simulink model "two_adcs_rfsoc_if_calibrator"  (R2021a)
settings: Solver=VariableStepAuto  StartTime=0.0  StopTime=10.0  RelTol=1e-3
undefined symbols (not defined in the file or in its folder): pfb_coeff_gen_calc
libraries: xbsIndex_r4 x30262, casper_library_misc x256, casper_library_delays x105, xps_library x1
(part 13 of 283)

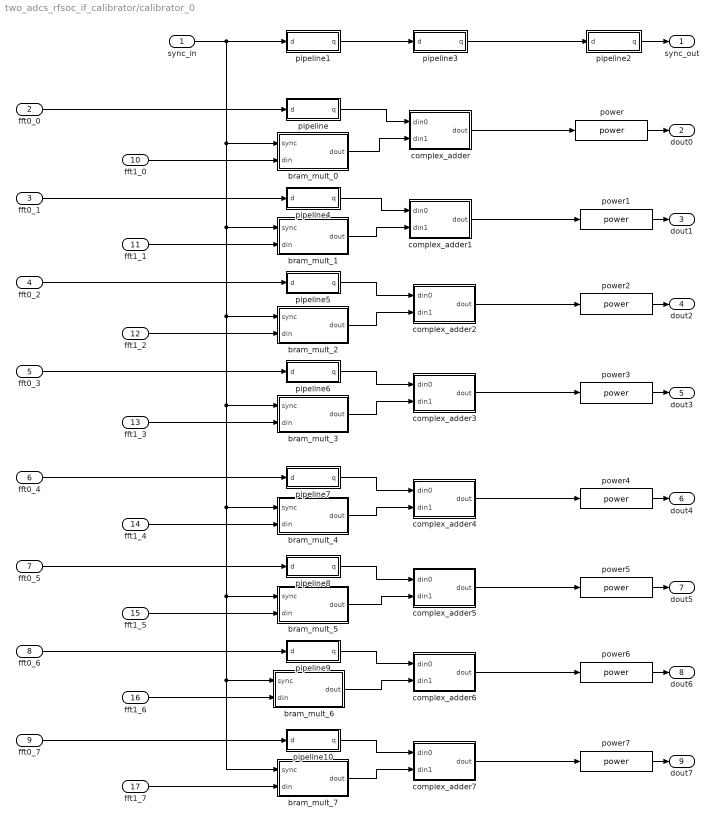
== system two_adcs_rfsoc_if_calibrator/calibrator_0 ==
  sync_in : Inport
  fft0_0 : Inport  Port=2
  fft0_1 : Inport  Port=3
  fft0_2 : Inport  Port=4
  fft0_3 : Inport  Port=5
  fft0_4 : Inport  Port=6
  fft0_5 : Inport  Port=7
  fft0_6 : Inport  Port=8
  fft0_7 : Inport  Port=9
  fft1_0 : Inport  Port=10
  fft1_1 : Inport  Port=11
  fft1_2 : Inport  Port=12
  fft1_3 : Inport  Port=13
  fft1_4 : Inport  Port=14
  fft1_5 : Inport  Port=15
  fft1_6 : Inport  Port=16
  fft1_7 : Inport  Port=17
  bram_mult_0 : Subsystem  mask{din_width=din_width, din_point=din_point, bram_width=bram_width, bram_point=bram_point, log2_vec_len=log2_vec_len, dout_width=din_width, dout_point=din_point}
      mask prompts: din_width "din width"; din_point "din point"; bram_width "bram width"; log2_vec_len "vector len (2?)"; dout_width "dout width"; dout_point "dout point"
      mask init:
      | name = gcb();
      | bram_name = strcat(name, '/bram_re');
      | set_param(bram_name, 'data_width', int2str(bram_width));
      | bram_name = strcat(name, '/bram_im');
      | set_param(bram_name, 'data_width', int2str(bram_width));
      mask Display:
      | port_label('input', 1, 'sync');
      | port_label('input', 2, 'din');
      | port_label('output', 1, 'dout');
      | disp('Z^-5')
  bram_mult_1 : Subsystem  mask{din_width=din_width, din_point=din_point, bram_width=bram_width, bram_point=bram_point, log2_vec_len=log2_vec_len, dout_width=din_width, dout_point=din_point}
      mask prompts: din_width "din width"; din_point "din point"; bram_width "bram width"; log2_vec_len "vector len (2?)"; dout_width "dout width"; dout_point "dout point"
      mask init: same as two_adcs_rfsoc_if_calibrator/calibrator_0/bram_mult_0
      mask Display:
      | port_label('input', 1, 'sync');
      | port_label('input', 2, 'din');
      | port_label('output', 1, 'dout');
      | disp('Z^-5')
  bram_mult_2 : Subsystem  mask{din_width=din_width, din_point=din_point, bram_width=bram_width, bram_point=bram_point, log2_vec_len=log2_vec_len, dout_width=din_width, dout_point=din_point}
      mask prompts: din_width "din width"; din_point "din point"; bram_width "bram width"; log2_vec_len "vector len (2?)"; dout_width "dout width"; dout_point "dout point"
      mask init: same as two_adcs_rfsoc_if_calibrator/calibrator_0/bram_mult_0
      mask Display:
      | port_label('input', 1, 'sync');
      | port_label('input', 2, 'din');
      | port_label('output', 1, 'dout');
      | disp('Z^-5')
  bram_mult_3 : Subsystem  mask{din_width=din_width, din_point=din_point, bram_width=bram_width, bram_point=bram_point, log2_vec_len=log2_vec_len, dout_width=din_width, dout_point=din_point}
      mask prompts: din_width "din width"; din_point "din point"; bram_width "bram width"; log2_vec_len "vector len (2?)"; dout_width "dout width"; dout_point "dout point"
      mask init: same as two_adcs_rfsoc_if_calibrator/calibrator_0/bram_mult_0
      mask Display:
      | port_label('input', 1, 'sync');
      | port_label('input', 2, 'din');
      | port_label('output', 1, 'dout');
      | disp('Z^-5')
  bram_mult_4 : Subsystem  mask{din_width=din_width, din_point=din_point, bram_width=bram_width, bram_point=bram_point, log2_vec_len=log2_vec_len, dout_width=din_width, dout_point=din_point}
      mask prompts: din_width "din width"; din_point "din point"; bram_width "bram width"; log2_vec_len "vector len (2?)"; dout_width "dout width"; dout_point "dout point"
      mask init: same as two_adcs_rfsoc_if_calibrator/calibrator_0/bram_mult_0
      mask Display:
      | port_label('input', 1, 'sync');
      | port_label('input', 2, 'din');
      | port_label('output', 1, 'dout');
      | disp('Z^-5')
  bram_mult_5 : Subsystem  mask{din_width=din_width, din_point=din_point, bram_width=bram_width, bram_point=bram_point, log2_vec_len=log2_vec_len, dout_width=din_width, dout_point=din_point}
      mask prompts: din_width "din width"; din_point "din point"; bram_width "bram width"; log2_vec_len "vector len (2?)"; dout_width "dout width"; dout_point "dout point"
      mask init: same as two_adcs_rfsoc_if_calibrator/calibrator_0/bram_mult_0
      mask Display:
      | port_label('input', 1, 'sync');
      | port_label('input', 2, 'din');
      | port_label('output', 1, 'dout');
      | disp('Z^-5')
  bram_mult_6 : Subsystem  mask{din_width=din_width, din_point=din_point, bram_width=bram_width, bram_point=bram_point, log2_vec_len=log2_vec_len, dout_width=din_width, dout_point=din_point}
      mask prompts: din_width "din width"; din_point "din point"; bram_width "bram width"; log2_vec_len "vector len (2?)"; dout_width "dout width"; dout_point "dout point"
      mask init: same as two_adcs_rfsoc_if_calibrator/calibrator_0/bram_mult_0
      mask Display:
      | port_label('input', 1, 'sync');
      | port_label('input', 2, 'din');
      | port_label('output', 1, 'dout');
      | disp('Z^-5')
  bram_mult_7 : Subsystem  mask{din_width=din_width, din_point=din_point, bram_width=bram_width, bram_point=bram_point, log2_vec_len=log2_vec_len, dout_width=din_width, dout_point=din_point}
      mask prompts: din_width "din width"; din_point "din point"; bram_width "bram width"; log2_vec_len "vector len (2?)"; dout_width "dout width"; dout_point "dout point"
      mask init: same as two_adcs_rfsoc_if_calibrator/calibrator_0/bram_mult_0
      mask Display:
      | port_label('input', 1, 'sync');
      | port_label('input', 2, 'din');
      | port_label('output', 1, 'dout');
      | disp('Z^-5')
  complex_adder : Subsystem  mask{Parameter1=din_width, din_point=din_point, dout_width=din_width, dout_point=din_point, add_lat=2, conv_lat=1}
      mask prompts: Parameter1 "din width"; din_point "din point"; dout_width "dout width"; dout_point "dout point"; add_lat "add delay"; conv_lat "conv delay"
  complex_adder1 : Subsystem  mask{Parameter1=din_width, din_point=din_point, dout_width=din_width, dout_point=din_point, add_lat=2, conv_lat=1}
      mask prompts: Parameter1 "din width"; din_point "din point"; dout_width "dout width"; dout_point "dout point"; add_lat "add delay"; conv_lat "conv delay"
  complex_adder2 : Subsystem  mask{Parameter1=din_width, din_point=din_point, dout_width=din_width, dout_point=din_point, add_lat=2, conv_lat=1}
      mask prompts: Parameter1 "din width"; din_point "din point"; dout_width "dout width"; dout_point "dout point"; add_lat "add delay"; conv_lat "conv delay"
  complex_adder3 : Subsystem  mask{Parameter1=din_width, din_point=din_point, dout_width=din_width, dout_point=din_point, add_lat=2, conv_lat=1}
      mask prompts: Parameter1 "din width"; din_point "din point"; dout_width "dout width"; dout_point "dout point"; add_lat "add delay"; conv_lat "conv delay"
  complex_adder4 : Subsystem  mask{Parameter1=din_width, din_point=din_point, dout_width=din_width, dout_point=din_point, add_lat=2, conv_lat=1}
      mask prompts: Parameter1 "din width"; din_point "din point"; dout_width "dout width"; dout_point "dout point"; add_lat "add delay"; conv_lat "conv delay"
  complex_adder5 : Subsystem  mask{Parameter1=din_width, din_point=din_point, dout_width=din_width, dout_point=din_point, add_lat=2, conv_lat=1}
      mask prompts: Parameter1 "din width"; din_point "din point"; dout_width "dout width"; dout_point "dout point"; add_lat "add delay"; conv_lat "conv delay"
  complex_adder6 : Subsystem  mask{Parameter1=din_width, din_point=din_point, dout_width=din_width, dout_point=din_point, add_lat=2, conv_lat=1}
      mask prompts: Parameter1 "din width"; din_point "din point"; dout_width "dout width"; dout_point "dout point"; add_lat "add delay"; conv_lat "conv delay"
  complex_adder7 : Subsystem  mask{Parameter1=din_width, din_point=din_point, dout_width=din_width, dout_point=din_point, add_lat=2, conv_lat=1}
      mask prompts: Parameter1 "din width"; din_point "din point"; dout_width "dout width"; dout_point "dout point"; add_lat "add delay"; conv_lat "conv delay"
  pipeline : Subsystem  AncestorBlock=casper_library_delays/pipeline  mask(pipeline){csp_latency=6}
      mask prompts: csp_latency "Latency"
      mask help:
      | An explicitly laid-out delay line to avoid mapping to SRL16s
      | for use in pipelining to help achieve timing closure.
      mask init:
      | pipeline_init(gcb,'latency',csp_latency);
      settings: AttributesFormatString=Latency = %<latency>
      mask Display:
      | port_label('input', 1, 'd');
      | port_label('output', 1, 'q');
      | disp(['Z^-', num2str(csp_latency)]);
  pipeline1 : Subsystem  AncestorBlock=casper_library_delays/pipeline  mask(pipeline){csp_latency=6}
      mask prompts: csp_latency "Latency"
      mask help:
      | An explicitly laid-out delay line to avoid mapping to SRL16s
      | for use in pipelining to help achieve timing closure.
      mask init:
      | pipeline_init(gcb,'latency',csp_latency);
      settings: AttributesFormatString=Latency = %<latency>
      mask Display:
      | port_label('input', 1, 'd');
      | port_label('output', 1, 'q');
      | disp(['Z^-', num2str(csp_latency)]);
  pipeline10 : Subsystem  AncestorBlock=casper_library_delays/pipeline  mask(pipeline){csp_latency=6}
      mask prompts: csp_latency "Latency"
      mask help:
      | An explicitly laid-out delay line to avoid mapping to SRL16s
      | for use in pipelining to help achieve timing closure.
      mask init:
      | pipeline_init(gcb,'latency',csp_latency);
      settings: AttributesFormatString=Latency = %<latency>
      mask Display:
      | port_label('input', 1, 'd');
      | port_label('output', 1, 'q');
      | disp(['Z^-', num2str(csp_latency)]);
  pipeline2 : Subsystem  AncestorBlock=casper_library_delays/pipeline  mask(pipeline){csp_latency=5}
      mask prompts: csp_latency "Latency"
      mask help:
      | An explicitly laid-out delay line to avoid mapping to SRL16s
      | for use in pipelining to help achieve timing closure.
      mask init:
      | pipeline_init(gcb,'latency',csp_latency);
      settings: AttributesFormatString=Latency = %<latency>
      mask Display:
      | port_label('input', 1, 'd');
      | port_label('output', 1, 'q');
      | disp(['Z^-', num2str(csp_latency)]);
  pipeline3 : Subsystem  AncestorBlock=casper_library_delays/pipeline  mask(pipeline){csp_latency=3}
      mask prompts: csp_latency "Latency"
      mask help:
      | An explicitly laid-out delay line to avoid mapping to SRL16s
      | for use in pipelining to help achieve timing closure.
      mask init:
      | pipeline_init(gcb,'latency',csp_latency);
      settings: AttributesFormatString=Latency = %<latency>
      mask Display:
      | port_label('input', 1, 'd');
      | port_label('output', 1, 'q');
      | disp(['Z^-', num2str(csp_latency)]);
  pipeline4 : Subsystem  AncestorBlock=casper_library_delays/pipeline  mask(pipeline){csp_latency=6}
      mask prompts: csp_latency "Latency"
      mask help:
      | An explicitly laid-out delay line to avoid mapping to SRL16s
      | for use in pipelining to help achieve timing closure.
      mask init:
      | pipeline_init(gcb,'latency',csp_latency);
      settings: AttributesFormatString=Latency = %<latency>
      mask Display:
      | port_label('input', 1, 'd');
      | port_label('output', 1, 'q');
      | disp(['Z^-', num2str(csp_latency)]);
  pipeline5 : Subsystem  AncestorBlock=casper_library_delays/pipeline  mask(pipeline){csp_latency=6}
      mask prompts: csp_latency "Latency"
      mask help:
      | An explicitly laid-out delay line to avoid mapping to SRL16s
      | for use in pipelining to help achieve timing closure.
      mask init:
      | pipeline_init(gcb,'latency',csp_latency);
      settings: AttributesFormatString=Latency = %<latency>
      mask Display:
      | port_label('input', 1, 'd');
      | port_label('output', 1, 'q');
      | disp(['Z^-', num2str(csp_latency)]);
  pipeline6 : Subsystem  AncestorBlock=casper_library_delays/pipeline  mask(pipeline){csp_latency=6}
      mask prompts: csp_latency "Latency"
      mask help:
      | An explicitly laid-out delay line to avoid mapping to SRL16s
      | for use in pipelining to help achieve timing closure.
      mask init:
      | pipeline_init(gcb,'latency',csp_latency);
      settings: AttributesFormatString=Latency = %<latency>
      mask Display:
      | port_label('input', 1, 'd');
      | port_label('output', 1, 'q');
      | disp(['Z^-', num2str(csp_latency)]);
  pipeline7 : Subsystem  AncestorBlock=casper_library_delays/pipeline  mask(pipeline){csp_latency=6}
      mask prompts: csp_latency "Latency"
      mask help:
      | An explicitly laid-out delay line to avoid mapping to SRL16s
      | for use in pipelining to help achieve timing closure.
      mask init:
      | pipeline_init(gcb,'latency',csp_latency);
      settings: AttributesFormatString=Latency = %<latency>
      mask Display:
      | port_label('input', 1, 'd');
      | port_label('output', 1, 'q');
      | disp(['Z^-', num2str(csp_latency)]);
  pipeline8 : Subsystem  AncestorBlock=casper_library_delays/pipeline  mask(pipeline){csp_latency=6}
      mask prompts: csp_latency "Latency"
      mask help:
      | An explicitly laid-out delay line to avoid mapping to SRL16s
      | for use in pipelining to help achieve timing closure.
      mask init:
      | pipeline_init(gcb,'latency',csp_latency);
      settings: AttributesFormatString=Latency = %<latency>
      mask Display:
      | port_label('input', 1, 'd');
      | port_label('output', 1, 'q');
      | disp(['Z^-', num2str(csp_latency)]);
  pipeline9 : Subsystem  AncestorBlock=casper_library_delays/pipeline  mask(pipeline){csp_latency=6}
      mask prompts: csp_latency "Latency"
      mask help:
      | An explicitly laid-out delay line to avoid mapping to SRL16s
      | for use in pipelining to help achieve timing closure.
      mask init:
      | pipeline_init(gcb,'latency',csp_latency);
      settings: AttributesFormatString=Latency = %<latency>
      mask Display:
      | port_label('input', 1, 'd');
      | port_label('output', 1, 'q');
      | disp(['Z^-', num2str(csp_latency)]);
  power{,1..7} (8 alike) : casper_library_misc/power  BitWidth=din_width  add_latency=2  mult_latency=3
      settings: SourceProductName=CASPER DSP Blockset
  sync_out : Outport
  dout0 : Outport  Port=2
  dout1 : Outport  Port=3
  dout2 : Outport  Port=4
  dout3 : Outport  Port=5
  dout4 : Outport  Port=6
  dout5 : Outport  Port=7
  dout6 : Outport  Port=8
  dout7 : Outport  Port=9
  wires:
  pipeline/1(q) -> complex_adder/1(din0)
  power/1 -> dout0/1
  fft0_0/1 -> pipeline/1(d)
  complex_adder/1(dout) -> power/1
  fft1_0/1 -> bram_mult_0/2(din)
  bram_mult_0/1(dout) -> complex_adder/2(din1)
  pipeline1/1(q) -> pipeline3/1(d)
  pipeline3/1(q) -> pipeline2/1(d)
  pipeline2/1(q) -> sync_out/1
  fft0_1/1 -> pipeline4/1(d)
  pipeline4/1(q) -> complex_adder1/1(din0)
  power1/1 -> dout1/1
  bram_mult_1/1(dout) -> complex_adder1/2(din1)
  bram_mult_3/1(dout) -> complex_adder3/2(din1)
  pipeline6/1(q) -> complex_adder3/1(din0)
  complex_adder2/1(dout) -> power2/1
  bram_mult_2/1(dout) -> complex_adder2/2(din1)
  fft0_3/1 -> pipeline6/1(d)
  power2/1 -> dout2/1
  complex_adder3/1(dout) -> power3/1
  power3/1 -> dout3/1
  pipeline5/1(q) -> complex_adder2/1(din0)
  fft0_2/1 -> pipeline5/1(d)
  sync_in/1 -> pipeline1/1(d), bram_mult_3/1(sync), bram_mult_5/1(sync), bram_mult_7/1(sync), bram_mult_6/1(sync), bram_mult_4/1(sync), bram_mult_2/1(sync), bram_mult_1/1(sync), bram_mult_0/1(sync)
  bram_mult_6/1(dout) -> complex_adder6/2(din1)
  complex_adder6/1(dout) -> power6/1
  bram_mult_5/1(dout) -> complex_adder5/2(din1)
  power5/1 -> dout5/1
  power4/1 -> dout4/1
  power6/1 -> dout6/1
  fft0_6/1 -> pipeline9/1(d)
  complex_adder5/1(dout) -> power5/1
  complex_adder4/1(dout) -> power4/1
  pipeline10/1(q) -> complex_adder7/1(din0)
  power7/1 -> dout7/1
  bram_mult_4/1(dout) -> complex_adder4/2(din1)
  pipeline8/1(q) -> complex_adder5/1(din0)
  fft0_4/1 -> pipeline7/1(d)
  bram_mult_7/1(dout) -> complex_adder7/2(din1)
  complex_adder7/1(dout) -> power7/1
  fft0_5/1 -> pipeline8/1(d)
  pipeline9/1(q) -> complex_adder6/1(din0)
  fft0_7/1 -> pipeline10/1(d)
  pipeline7/1(q) -> complex_adder4/1(din0)
  fft1_1/1 -> bram_mult_1/2(din)
  fft1_2/1 -> bram_mult_2/2(din)
  fft1_3/1 -> bram_mult_3/2(din)
  fft1_4/1 -> bram_mult_4/2(din)
  fft1_5/1 -> bram_mult_5/2(din)
  fft1_6/1 -> bram_mult_6/2(din)
  fft1_7/1 -> bram_mult_7/2(din)
  complex_adder1/1(dout) -> power1/1

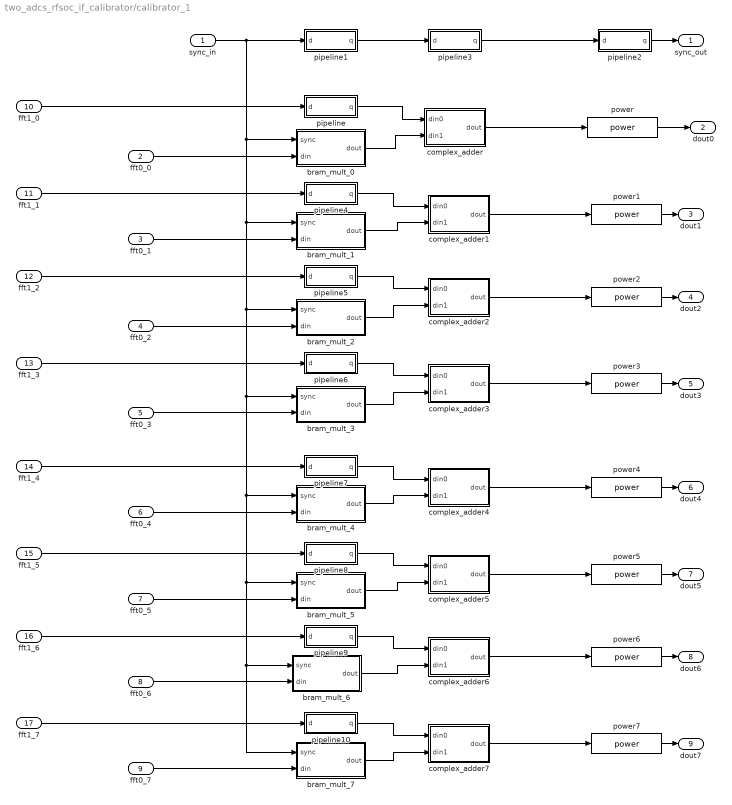
== system two_adcs_rfsoc_if_calibrator/calibrator_1 ==
  sync_in : Inport
  fft0_0 : Inport  Port=2
  fft0_1 : Inport  Port=3
  fft0_2 : Inport  Port=4
  fft0_3 : Inport  Port=5
  fft0_4 : Inport  Port=6
  fft0_5 : Inport  Port=7
  fft0_6 : Inport  Port=8
  fft0_7 : Inport  Port=9
  fft1_0 : Inport  Port=10
  fft1_1 : Inport  Port=11
  fft1_2 : Inport  Port=12
  fft1_3 : Inport  Port=13
  fft1_4 : Inport  Port=14
  fft1_5 : Inport  Port=15
  fft1_6 : Inport  Port=16
  fft1_7 : Inport  Port=17
  bram_mult_0 : Subsystem  mask{din_width=din_width, din_point=din_point, bram_width=bram_width, bram_point=bram_point, log2_vec_len=log2_vec_len, dout_width=din_width, dout_point=din_point}
      mask prompts: din_width "din width"; din_point "din point"; bram_width "bram width"; log2_vec_len "vector len (2?)"; dout_width "dout width"; dout_point "dout point"
      mask init: same as two_adcs_rfsoc_if_calibrator/calibrator_0/bram_mult_0
      mask Display:
      | port_label('input', 1, 'sync');
      | port_label('input', 2, 'din');
      | port_label('output', 1, 'dout');
      | disp('Z^-5')
  bram_mult_1 : Subsystem  mask{din_width=din_width, din_point=din_point, bram_width=bram_width, bram_point=bram_point, log2_vec_len=log2_vec_len, dout_width=din_width, dout_point=din_point}
      mask prompts: din_width "din width"; din_point "din point"; bram_width "bram width"; log2_vec_len "vector len (2?)"; dout_width "dout width"; dout_point "dout point"
      mask init: same as two_adcs_rfsoc_if_calibrator/calibrator_0/bram_mult_0
      mask Display:
      | port_label('input', 1, 'sync');
      | port_label('input', 2, 'din');
      | port_label('output', 1, 'dout');
      | disp('Z^-5')
  bram_mult_2 : Subsystem  mask{din_width=din_width, din_point=din_point, bram_width=bram_width, bram_point=bram_point, log2_vec_len=log2_vec_len, dout_width=din_width, dout_point=din_point}
      mask prompts: din_width "din width"; din_point "din point"; bram_width "bram width"; log2_vec_len "vector len (2?)"; dout_width "dout width"; dout_point "dout point"
      mask init: same as two_adcs_rfsoc_if_calibrator/calibrator_0/bram_mult_0
      mask Display:
      | port_label('input', 1, 'sync');
      | port_label('input', 2, 'din');
      | port_label('output', 1, 'dout');
      | disp('Z^-5')
  bram_mult_3 : Subsystem  mask{din_width=din_width, din_point=din_point, bram_width=bram_width, bram_point=bram_point, log2_vec_len=log2_vec_len, dout_width=din_width, dout_point=din_point}
      mask prompts: din_width "din width"; din_point "din point"; bram_width "bram width"; log2_vec_len "vector len (2?)"; dout_width "dout width"; dout_point "dout point"
      mask init: same as two_adcs_rfsoc_if_calibrator/calibrator_0/bram_mult_0
      mask Display:
      | port_label('input', 1, 'sync');
      | port_label('input', 2, 'din');
      | port_label('output', 1, 'dout');
      | disp('Z^-5')
  bram_mult_4 : Subsystem  mask{din_width=din_width, din_point=din_point, bram_width=bram_width, bram_point=bram_point, log2_vec_len=log2_vec_len, dout_width=din_width, dout_point=din_point}
      mask prompts: din_width "din width"; din_point "din point"; bram_width "bram width"; log2_vec_len "vector len (2?)"; dout_width "dout width"; dout_point "dout point"
      mask init: same as two_adcs_rfsoc_if_calibrator/calibrator_0/bram_mult_0
      mask Display:
      | port_label('input', 1, 'sync');
      | port_label('input', 2, 'din');
      | port_label('output', 1, 'dout');
      | disp('Z^-5')
  bram_mult_5 : Subsystem  mask{din_width=din_width, din_point=din_point, bram_width=bram_width, bram_point=bram_point, log2_vec_len=log2_vec_len, dout_width=din_width, dout_point=din_point}
      mask prompts: din_width "din width"; din_point "din point"; bram_width "bram width"; log2_vec_len "vector len (2?)"; dout_width "dout width"; dout_point "dout point"
      mask init: same as two_adcs_rfsoc_if_calibrator/calibrator_0/bram_mult_0
      mask Display:
      | port_label('input', 1, 'sync');
      | port_label('input', 2, 'din');
      | port_label('output', 1, 'dout');
      | disp('Z^-5')
  bram_mult_6 : Subsystem  mask{din_width=din_width, din_point=din_point, bram_width=bram_width, bram_point=bram_point, log2_vec_len=log2_vec_len, dout_width=din_width, dout_point=din_point}
      mask prompts: din_width "din width"; din_point "din point"; bram_width "bram width"; log2_vec_len "vector len (2?)"; dout_width "dout width"; dout_point "dout point"
      mask init: same as two_adcs_rfsoc_if_calibrator/calibrator_0/bram_mult_0
      mask Display:
      | port_label('input', 1, 'sync');
      | port_label('input', 2, 'din');
      | port_label('output', 1, 'dout');
      | disp('Z^-5')
  bram_mult_7 : Subsystem  mask{din_width=din_width, din_point=din_point, bram_width=bram_width, bram_point=bram_point, log2_vec_len=log2_vec_len, dout_width=din_width, dout_point=din_point}
      mask prompts: din_width "din width"; din_point "din point"; bram_width "bram width"; log2_vec_len "vector len (2?)"; dout_width "dout width"; dout_point "dout point"
      mask init: same as two_adcs_rfsoc_if_calibrator/calibrator_0/bram_mult_0
      mask Display:
      | port_label('input', 1, 'sync');
      | port_label('input', 2, 'din');
      | port_label('output', 1, 'dout');
      | disp('Z^-5')
  complex_adder : Subsystem  mask{Parameter1=din_width, din_point=din_point, dout_width=din_width, dout_point=din_point, add_lat=2, conv_lat=1}
      mask prompts: Parameter1 "din width"; din_point "din point"; dout_width "dout width"; dout_point "dout point"; add_lat "add delay"; conv_lat "conv delay"
  complex_adder1 : Subsystem  mask{Parameter1=din_width, din_point=din_point, dout_width=din_width, dout_point=din_point, add_lat=2, conv_lat=1}
      mask prompts: Parameter1 "din width"; din_point "din point"; dout_width "dout width"; dout_point "dout point"; add_lat "add delay"; conv_lat "conv delay"
  complex_adder2 : Subsystem  mask{Parameter1=din_width, din_point=din_point, dout_width=din_width, dout_point=din_point, add_lat=2, conv_lat=1}
      mask prompts: Parameter1 "din width"; din_point "din point"; dout_width "dout width"; dout_point "dout point"; add_lat "add delay"; conv_lat "conv delay"
  complex_adder3 : Subsystem  mask{Parameter1=din_width, din_point=din_point, dout_width=din_width, dout_point=din_point, add_lat=2, conv_lat=1}
      mask prompts: Parameter1 "din width"; din_point "din point"; dout_width "dout width"; dout_point "dout point"; add_lat "add delay"; conv_lat "conv delay"
  complex_adder4 : Subsystem  mask{Parameter1=din_width, din_point=din_point, dout_width=din_width, dout_point=din_point, add_lat=2, conv_lat=1}
      mask prompts: Parameter1 "din width"; din_point "din point"; dout_width "dout width"; dout_point "dout point"; add_lat "add delay"; conv_lat "conv delay"
  complex_adder5 : Subsystem  mask{Parameter1=din_width, din_point=din_point, dout_width=din_width, dout_point=din_point, add_lat=2, conv_lat=1}
      mask prompts: Parameter1 "din width"; din_point "din point"; dout_width "dout width"; dout_point "dout point"; add_lat "add delay"; conv_lat "conv delay"
  complex_adder6 : Subsystem  mask{Parameter1=din_width, din_point=din_point, dout_width=din_width, dout_point=din_point, add_lat=2, conv_lat=1}
      mask prompts: Parameter1 "din width"; din_point "din point"; dout_width "dout width"; dout_point "dout point"; add_lat "add delay"; conv_lat "conv delay"
  complex_adder7 : Subsystem  mask{Parameter1=din_width, din_point=din_point, dout_width=din_width, dout_point=din_point, add_lat=2, conv_lat=1}
      mask prompts: Parameter1 "din width"; din_point "din point"; dout_width "dout width"; dout_point "dout point"; add_lat "add delay"; conv_lat "conv delay"
  pipeline : Subsystem  AncestorBlock=casper_library_delays/pipeline  mask(pipeline){csp_latency=6}
      mask prompts: csp_latency "Latency"
      mask help:
      | An explicitly laid-out delay line to avoid mapping to SRL16s
      | for use in pipelining to help achieve timing closure.
      mask init:
      | pipeline_init(gcb,'latency',csp_latency);
      settings: AttributesFormatString=Latency = %<latency>
      mask Display:
      | port_label('input', 1, 'd');
      | port_label('output', 1, 'q');
      | disp(['Z^-', num2str(csp_latency)]);
  pipeline1 : Subsystem  AncestorBlock=casper_library_delays/pipeline  mask(pipeline){csp_latency=6}
      mask prompts: csp_latency "Latency"
      mask help:
      | An explicitly laid-out delay line to avoid mapping to SRL16s
      | for use in pipelining to help achieve timing closure.
      mask init:
      | pipeline_init(gcb,'latency',csp_latency);
      settings: AttributesFormatString=Latency = %<latency>
      mask Display:
      | port_label('input', 1, 'd');
      | port_label('output', 1, 'q');
      | disp(['Z^-', num2str(csp_latency)]);
  pipeline10 : Subsystem  AncestorBlock=casper_library_delays/pipeline  mask(pipeline){csp_latency=6}
      mask prompts: csp_latency "Latency"
      mask help:
      | An explicitly laid-out delay line to avoid mapping to SRL16s
      | for use in pipelining to help achieve timing closure.
      mask init:
      | pipeline_init(gcb,'latency',csp_latency);
      settings: AttributesFormatString=Latency = %<latency>
      mask Display:
      | port_label('input', 1, 'd');
      | port_label('output', 1, 'q');
      | disp(['Z^-', num2str(csp_latency)]);
  pipeline2 : Subsystem  AncestorBlock=casper_library_delays/pipeline  mask(pipeline){csp_latency=5}
      mask prompts: csp_latency "Latency"
      mask help:
      | An explicitly laid-out delay line to avoid mapping to SRL16s
      | for use in pipelining to help achieve timing closure.
      mask init:
      | pipeline_init(gcb,'latency',csp_latency);
      settings: AttributesFormatString=Latency = %<latency>
      mask Display:
      | port_label('input', 1, 'd');
      | port_label('output', 1, 'q');
      | disp(['Z^-', num2str(csp_latency)]);
  pipeline3 : Subsystem  AncestorBlock=casper_library_delays/pipeline  mask(pipeline){csp_latency=3}
      mask prompts: csp_latency "Latency"
      mask help:
      | An explicitly laid-out delay line to avoid mapping to SRL16s
      | for use in pipelining to help achieve timing closure.
      mask init:
      | pipeline_init(gcb,'latency',csp_latency);
      settings: AttributesFormatString=Latency = %<latency>
      mask Display:
      | port_label('input', 1, 'd');
      | port_label('output', 1, 'q');
      | disp(['Z^-', num2str(csp_latency)]);
  pipeline4 : Subsystem  AncestorBlock=casper_library_delays/pipeline  mask(pipeline){csp_latency=6}
      mask prompts: csp_latency "Latency"
      mask help:
      | An explicitly laid-out delay line to avoid mapping to SRL16s
      | for use in pipelining to help achieve timing closure.
      mask init:
      | pipeline_init(gcb,'latency',csp_latency);
      settings: AttributesFormatString=Latency = %<latency>
      mask Display:
      | port_label('input', 1, 'd');
      | port_label('output', 1, 'q');
      | disp(['Z^-', num2str(csp_latency)]);
  pipeline5 : Subsystem  AncestorBlock=casper_library_delays/pipeline  mask(pipeline){csp_latency=6}
      mask prompts: csp_latency "Latency"
      mask help:
      | An explicitly laid-out delay line to avoid mapping to SRL16s
      | for use in pipelining to help achieve timing closure.
      mask init:
      | pipeline_init(gcb,'latency',csp_latency);
      settings: AttributesFormatString=Latency = %<latency>
      mask Display:
      | port_label('input', 1, 'd');
      | port_label('output', 1, 'q');
      | disp(['Z^-', num2str(csp_latency)]);
  pipeline6 : Subsystem  AncestorBlock=casper_library_delays/pipeline  mask(pipeline){csp_latency=6}
      mask prompts: csp_latency "Latency"
      mask help:
      | An explicitly laid-out delay line to avoid mapping to SRL16s
      | for use in pipelining to help achieve timing closure.
      mask init:
      | pipeline_init(gcb,'latency',csp_latency);
      settings: AttributesFormatString=Latency = %<latency>
      mask Display:
      | port_label('input', 1, 'd');
      | port_label('output', 1, 'q');
      | disp(['Z^-', num2str(csp_latency)]);
  pipeline7 : Subsystem  AncestorBlock=casper_library_delays/pipeline  mask(pipeline){csp_latency=6}
      mask prompts: csp_latency "Latency"
      mask help:
      | An explicitly laid-out delay line to avoid mapping to SRL16s
      | for use in pipelining to help achieve timing closure.
      mask init:
      | pipeline_init(gcb,'latency',csp_latency);
      settings: AttributesFormatString=Latency = %<latency>
      mask Display:
      | port_label('input', 1, 'd');
      | port_label('output', 1, 'q');
      | disp(['Z^-', num2str(csp_latency)]);
  pipeline8 : Subsystem  AncestorBlock=casper_library_delays/pipeline  mask(pipeline){csp_latency=6}
      mask prompts: csp_latency "Latency"
      mask help:
      | An explicitly laid-out delay line to avoid mapping to SRL16s
      | for use in pipelining to help achieve timing closure.
      mask init:
      | pipeline_init(gcb,'latency',csp_latency);
      settings: AttributesFormatString=Latency = %<latency>
      mask Display:
      | port_label('input', 1, 'd');
      | port_label('output', 1, 'q');
      | disp(['Z^-', num2str(csp_latency)]);
  pipeline9 : Subsystem  AncestorBlock=casper_library_delays/pipeline  mask(pipeline){csp_latency=6}
      mask prompts: csp_latency "Latency"
      mask help:
      | An explicitly laid-out delay line to avoid mapping to SRL16s
      | for use in pipelining to help achieve timing closure.
      mask init:
      | pipeline_init(gcb,'latency',csp_latency);
      settings: AttributesFormatString=Latency = %<latency>
      mask Display:
      | port_label('input', 1, 'd');
      | port_label('output', 1, 'q');
      | disp(['Z^-', num2str(csp_latency)]);
  power{,1..7} (8 alike) : casper_library_misc/power  BitWidth=din_width  add_latency=2  mult_latency=3
      settings: SourceProductName=CASPER DSP Blockset
  sync_out : Outport
  dout0 : Outport  Port=2
  dout1 : Outport  Port=3
  dout2 : Outport  Port=4
  dout3 : Outport  Port=5
  dout4 : Outport  Port=6
  dout5 : Outport  Port=7
  dout6 : Outport  Port=8
  dout7 : Outport  Port=9
  wires:
  pipeline/1(q) -> complex_adder/1(din0)
  power/1 -> dout0/1
  complex_adder/1(dout) -> power/1
  bram_mult_0/1(dout) -> complex_adder/2(din1)
  pipeline1/1(q) -> pipeline3/1(d)
  pipeline3/1(q) -> pipeline2/1(d)
  pipeline2/1(q) -> sync_out/1
  pipeline4/1(q) -> complex_adder1/1(din0)
  power1/1 -> dout1/1
  bram_mult_1/1(dout) -> complex_adder1/2(din1)
  bram_mult_3/1(dout) -> complex_adder3/2(din1)
  pipeline6/1(q) -> complex_adder3/1(din0)
  complex_adder2/1(dout) -> power2/1
  bram_mult_2/1(dout) -> complex_adder2/2(din1)
  power2/1 -> dout2/1
  complex_adder3/1(dout) -> power3/1
  power3/1 -> dout3/1
  pipeline5/1(q) -> complex_adder2/1(din0)
  sync_in/1 -> pipeline1/1(d), bram_mult_3/1(sync), bram_mult_5/1(sync), bram_mult_7/1(sync), bram_mult_6/1(sync), bram_mult_4/1(sync), bram_mult_2/1(sync), bram_mult_1/1(sync), bram_mult_0/1(sync)
  bram_mult_6/1(dout) -> complex_adder6/2(din1)
  complex_adder6/1(dout) -> power6/1
  bram_mult_5/1(dout) -> complex_adder5/2(din1)
  power5/1 -> dout5/1
  power4/1 -> dout4/1
  power6/1 -> dout6/1
  complex_adder5/1(dout) -> power5/1
  complex_adder4/1(dout) -> power4/1
  pipeline10/1(q) -> complex_adder7/1(din0)
  power7/1 -> dout7/1
  bram_mult_4/1(dout) -> complex_adder4/2(din1)
  pipeline8/1(q) -> complex_adder5/1(din0)
  bram_mult_7/1(dout) -> complex_adder7/2(din1)
  complex_adder7/1(dout) -> power7/1
  pipeline9/1(q) -> complex_adder6/1(din0)
  pipeline7/1(q) -> complex_adder4/1(din0)
  complex_adder1/1(dout) -> power1/1
  fft0_0/1 -> bram_mult_0/2(din)
  fft1_0/1 -> pipeline/1(d)
  fft1_1/1 -> pipeline4/1(d)
  fft0_1/1 -> bram_mult_1/2(din)
  fft1_2/1 -> pipeline5/1(d)
  fft0_2/1 -> bram_mult_2/2(din)
  fft1_4/1 -> pipeline7/1(d)
  fft0_4/1 -> bram_mult_4/2(din)
  fft1_5/1 -> pipeline8/1(d)
  fft0_5/1 -> bram_mult_5/2(din)
  fft1_6/1 -> pipeline9/1(d)
  fft0_6/1 -> bram_mult_6/2(din)
  fft1_7/1 -> pipeline10/1(d)
  fft0_7/1 -> bram_mult_7/2(din)
  fft1_3/1 -> pipeline6/1(d)
  fft0_3/1 -> bram_mult_3/2(din)

== system two_adcs_rfsoc_if_calibrator/calibrator_accs0 ==
  new_acc : Inport
  din : Inport  Port=2
  din1 : Inport  Port=3
  din2 : Inport  Port=4
  din3 : Inport  Port=5
  din4 : Inport  Port=6
  din5 : Inport  Port=7
  din6 : Inport  Port=8
  din7 : Inport  Port=9
  simple_bram_vacc : Subsystem  AncestorBlock=casper_library_accumulators/simple_bram_vacc  mask(simple_bram_vacc){vec_len=vector_len, arith_type=Unsigned, n_bits=dout_width, bin_pt=dout_point}
      mask prompts: vec_len "Vector length:"; arith_type "Output type:"; n_bits "Bit width (output):"; bin_pt "Binary point (output):"
      mask help: This simple vector accumulator is based on a shift register. It outputs its previous accumulated vector after a new_acc pulse is received.
      mask init:
      | simple_bram_vacc_init(gcb, ...
      |     'vec_len', vec_len, ...
      |     'arith_type', arith_type, ...
      |     'n_bits', n_bits, ...
      |     'bin_pt', bin_pt);
      mask TypeOptions arith_type: Signed | Unsigned
  simple_bram_vacc1 : Subsystem  AncestorBlock=casper_library_accumulators/simple_bram_vacc  mask(simple_bram_vacc){vec_len=vector_len, arith_type=Unsigned, n_bits=dout_width, bin_pt=dout_point}
      mask prompts: vec_len "Vector length:"; arith_type "Output type:"; n_bits "Bit width (output):"; bin_pt "Binary point (output):"
      mask help: This simple vector accumulator is based on a shift register. It outputs its previous accumulated vector after a new_acc pulse is received.
      mask init:
      | simple_bram_vacc_init(gcb, ...
      |     'vec_len', vec_len, ...
      |     'arith_type', arith_type, ...
      |     'n_bits', n_bits, ...
      |     'bin_pt', bin_pt);
      mask TypeOptions arith_type: Signed | Unsigned
  simple_bram_vacc2 : Subsystem  AncestorBlock=casper_library_accumulators/simple_bram_vacc  mask(simple_bram_vacc){vec_len=vector_len, arith_type=Unsigned, n_bits=dout_width, bin_pt=dout_point}
      mask prompts: vec_len "Vector length:"; arith_type "Output type:"; n_bits "Bit width (output):"; bin_pt "Binary point (output):"
      mask help: This simple vector accumulator is based on a shift register. It outputs its previous accumulated vector after a new_acc pulse is received.
      mask init:
      | simple_bram_vacc_init(gcb, ...
      |     'vec_len', vec_len, ...
      |     'arith_type', arith_type, ...
      |     'n_bits', n_bits, ...
      |     'bin_pt', bin_pt);
      mask TypeOptions arith_type: Signed | Unsigned
  simple_bram_vacc3 : Subsystem  AncestorBlock=casper_library_accumulators/simple_bram_vacc  mask(simple_bram_vacc){vec_len=vector_len, arith_type=Unsigned, n_bits=dout_width, bin_pt=dout_point}
      mask prompts: vec_len "Vector length:"; arith_type "Output type:"; n_bits "Bit width (output):"; bin_pt "Binary point (output):"
      mask help: This simple vector accumulator is based on a shift register. It outputs its previous accumulated vector after a new_acc pulse is received.
      mask init:
      | simple_bram_vacc_init(gcb, ...
      |     'vec_len', vec_len, ...
      |     'arith_type', arith_type, ...
      |     'n_bits', n_bits, ...
      |     'bin_pt', bin_pt);
      mask TypeOptions arith_type: Signed | Unsigned
  simple_bram_vacc4 : Subsystem  AncestorBlock=casper_library_accumulators/simple_bram_vacc  mask(simple_bram_vacc){vec_len=vector_len, arith_type=Unsigned, n_bits=dout_width, bin_pt=dout_point}
      mask prompts: vec_len "Vector length:"; arith_type "Output type:"; n_bits "Bit width (output):"; bin_pt "Binary point (output):"
      mask help: This simple vector accumulator is based on a shift register. It outputs its previous accumulated vector after a new_acc pulse is received.
      mask init:
      | simple_bram_vacc_init(gcb, ...
      |     'vec_len', vec_len, ...
      |     'arith_type', arith_type, ...
      |     'n_bits', n_bits, ...
      |     'bin_pt', bin_pt);
      mask TypeOptions arith_type: Signed | Unsigned
  simple_bram_vacc5 : Subsystem  AncestorBlock=casper_library_accumulators/simple_bram_vacc  mask(simple_bram_vacc){vec_len=vector_len, arith_type=Unsigned, n_bits=dout_width, bin_pt=dout_point}
      mask prompts: vec_len "Vector length:"; arith_type "Output type:"; n_bits "Bit width (output):"; bin_pt "Binary point (output):"
      mask help: This simple vector accumulator is based on a shift register. It outputs its previous accumulated vector after a new_acc pulse is received.
      mask init:
      | simple_bram_vacc_init(gcb, ...
      |     'vec_len', vec_len, ...
      |     'arith_type', arith_type, ...
      |     'n_bits', n_bits, ...
      |     'bin_pt', bin_pt);
      mask TypeOptions arith_type: Signed | Unsigned
  simple_bram_vacc6 : Subsystem  AncestorBlock=casper_library_accumulators/simple_bram_vacc  mask(simple_bram_vacc){vec_len=vector_len, arith_type=Unsigned, n_bits=dout_width, bin_pt=dout_point}
      mask prompts: vec_len "Vector length:"; arith_type "Output type:"; n_bits "Bit width (output):"; bin_pt "Binary point (output):"
      mask help: This simple vector accumulator is based on a shift register. It outputs its previous accumulated vector after a new_acc pulse is received.
      mask init:
      | simple_bram_vacc_init(gcb, ...
      |     'vec_len', vec_len, ...
      |     'arith_type', arith_type, ...
      |     'n_bits', n_bits, ...
      |     'bin_pt', bin_pt);
      mask TypeOptions arith_type: Signed | Unsigned
  simple_bram_vacc7 : Subsystem  AncestorBlock=casper_library_accumulators/simple_bram_vacc  mask(simple_bram_vacc){vec_len=vector_len, arith_type=Unsigned, n_bits=dout_width, bin_pt=dout_point}
      mask prompts: vec_len "Vector length:"; arith_type "Output type:"; n_bits "Bit width (output):"; bin_pt "Binary point (output):"
      mask help: This simple vector accumulator is based on a shift register. It outputs its previous accumulated vector after a new_acc pulse is received.
      mask init:
      | simple_bram_vacc_init(gcb, ...
      |     'vec_len', vec_len, ...
      |     'arith_type', arith_type, ...
      |     'n_bits', n_bits, ...
      |     'bin_pt', bin_pt);
      mask TypeOptions arith_type: Signed | Unsigned
  valid : Outport
  dout0 : Outport  Port=2
  dout1 : Outport  Port=3
  dout2 : Outport  Port=4
  dout3 : Outport  Port=5
  dout4 : Outport  Port=6
  dout5 : Outport  Port=7
  dout6 : Outport  Port=8
  dout7 : Outport  Port=9
  wires:
  din/1 -> simple_bram_vacc/2(din)
  din3/1 -> simple_bram_vacc3/2(din)
  simple_bram_vacc3/2(valid) -> valid/1
  simple_bram_vacc1/1(dout) -> dout1/1
  simple_bram_vacc2/1(dout) -> dout2/1
  din2/1 -> simple_bram_vacc2/2(din)
  simple_bram_vacc3/1(dout) -> dout3/1
  simple_bram_vacc/1(dout) -> dout0/1
  din1/1 -> simple_bram_vacc1/2(din)
  simple_bram_vacc7/1(dout) -> dout7/1
  simple_bram_vacc6/1(dout) -> dout6/1
  simple_bram_vacc4/1(dout) -> dout4/1
  din7/1 -> simple_bram_vacc7/2(din)
  new_acc/1 -> simple_bram_vacc/1(new_acc), simple_bram_vacc1/1(new_acc), simple_bram_vacc4/1(new_acc), simple_bram_vacc5/1(new_acc), simple_bram_vacc7/1(new_acc), simple_bram_vacc6/1(new_acc), simple_bram_vacc3/1(new_acc), simple_bram_vacc2/1(new_acc)
  din4/1 -> simple_bram_vacc4/2(din)
  din5/1 -> simple_bram_vacc5/2(din)
  simple_bram_vacc5/1(dout) -> dout5/1
  din6/1 -> simple_bram_vacc6/2(din)

== system two_adcs_rfsoc_if_calibrator/calibrator_accs1 ==
  new_acc : Inport
  din : Inport  Port=2
  din1 : Inport  Port=3
  din2 : Inport  Port=4
  din3 : Inport  Port=5
  din4 : Inport  Port=6
  din5 : Inport  Port=7
  din6 : Inport  Port=8
  din7 : Inport  Port=9
  simple_bram_vacc : Subsystem  AncestorBlock=casper_library_accumulators/simple_bram_vacc  mask(simple_bram_vacc){vec_len=vector_len, arith_type=Unsigned, n_bits=dout_width, bin_pt=dout_point}
      mask prompts: vec_len "Vector length:"; arith_type "Output type:"; n_bits "Bit width (output):"; bin_pt "Binary point (output):"
      mask help: This simple vector accumulator is based on a shift register. It outputs its previous accumulated vector after a new_acc pulse is received.
      mask init:
      | simple_bram_vacc_init(gcb, ...
      |     'vec_len', vec_len, ...
      |     'arith_type', arith_type, ...
      |     'n_bits', n_bits, ...
      |     'bin_pt', bin_pt);
      mask TypeOptions arith_type: Signed | Unsigned
  simple_bram_vacc1 : Subsystem  AncestorBlock=casper_library_accumulators/simple_bram_vacc  mask(simple_bram_vacc){vec_len=vector_len, arith_type=Unsigned, n_bits=dout_width, bin_pt=dout_point}
      mask prompts: vec_len "Vector length:"; arith_type "Output type:"; n_bits "Bit width (output):"; bin_pt "Binary point (output):"
      mask help: This simple vector accumulator is based on a shift register. It outputs its previous accumulated vector after a new_acc pulse is received.
      mask init:
      | simple_bram_vacc_init(gcb, ...
      |     'vec_len', vec_len, ...
      |     'arith_type', arith_type, ...
      |     'n_bits', n_bits, ...
      |     'bin_pt', bin_pt);
      mask TypeOptions arith_type: Signed | Unsigned
  simple_bram_vacc2 : Subsystem  AncestorBlock=casper_library_accumulators/simple_bram_vacc  mask(simple_bram_vacc){vec_len=vector_len, arith_type=Unsigned, n_bits=dout_width, bin_pt=dout_point}
      mask prompts: vec_len "Vector length:"; arith_type "Output type:"; n_bits "Bit width (output):"; bin_pt "Binary point (output):"
      mask help: This simple vector accumulator is based on a shift register. It outputs its previous accumulated vector after a new_acc pulse is received.
      mask init:
      | simple_bram_vacc_init(gcb, ...
      |     'vec_len', vec_len, ...
      |     'arith_type', arith_type, ...
      |     'n_bits', n_bits, ...
      |     'bin_pt', bin_pt);
      mask TypeOptions arith_type: Signed | Unsigned
  simple_bram_vacc3 : Subsystem  AncestorBlock=casper_library_accumulators/simple_bram_vacc  mask(simple_bram_vacc){vec_len=vector_len, arith_type=Unsigned, n_bits=dout_width, bin_pt=dout_point}
      mask prompts: vec_len "Vector length:"; arith_type "Output type:"; n_bits "Bit width (output):"; bin_pt "Binary point (output):"
      mask help: This simple vector accumulator is based on a shift register. It outputs its previous accumulated vector after a new_acc pulse is received.
      mask init:
      | simple_bram_vacc_init(gcb, ...
      |     'vec_len', vec_len, ...
      |     'arith_type', arith_type, ...
      |     'n_bits', n_bits, ...
      |     'bin_pt', bin_pt);
      mask TypeOptions arith_type: Signed | Unsigned
  simple_bram_vacc4 : Subsystem  AncestorBlock=casper_library_accumulators/simple_bram_vacc  mask(simple_bram_vacc){vec_len=vector_len, arith_type=Unsigned, n_bits=dout_width, bin_pt=dout_point}
      mask prompts: vec_len "Vector length:"; arith_type "Output type:"; n_bits "Bit width (output):"; bin_pt "Binary point (output):"
      mask help: This simple vector accumulator is based on a shift register. It outputs its previous accumulated vector after a new_acc pulse is received.
      mask init:
      | simple_bram_vacc_init(gcb, ...
      |     'vec_len', vec_len, ...
      |     'arith_type', arith_type, ...
      |     'n_bits', n_bits, ...
      |     'bin_pt', bin_pt);
      mask TypeOptions arith_type: Signed | Unsigned
  simple_bram_vacc5 : Subsystem  AncestorBlock=casper_library_accumulators/simple_bram_vacc  mask(simple_bram_vacc){vec_len=vector_len, arith_type=Unsigned, n_bits=dout_width, bin_pt=dout_point}
      mask prompts: vec_len "Vector length:"; arith_type "Output type:"; n_bits "Bit width (output):"; bin_pt "Binary point (output):"
      mask help: This simple vector accumulator is based on a shift register. It outputs its previous accumulated vector after a new_acc pulse is received.
      mask init:
      | simple_bram_vacc_init(gcb, ...
      |     'vec_len', vec_len, ...
      |     'arith_type', arith_type, ...
      |     'n_bits', n_bits, ...
      |     'bin_pt', bin_pt);
      mask TypeOptions arith_type: Signed | Unsigned
  simple_bram_vacc6 : Subsystem  AncestorBlock=casper_library_accumulators/simple_bram_vacc  mask(simple_bram_vacc){vec_len=vector_len, arith_type=Unsigned, n_bits=dout_width, bin_pt=dout_point}
      mask prompts: vec_len "Vector length:"; arith_type "Output type:"; n_bits "Bit width (output):"; bin_pt "Binary point (output):"
      mask help: This simple vector accumulator is based on a shift register. It outputs its previous accumulated vector after a new_acc pulse is received.
      mask init:
      | simple_bram_vacc_init(gcb, ...
      |     'vec_len', vec_len, ...
      |     'arith_type', arith_type, ...
      |     'n_bits', n_bits, ...
      |     'bin_pt', bin_pt);
      mask TypeOptions arith_type: Signed | Unsigned
  simple_bram_vacc7 : Subsystem  AncestorBlock=casper_library_accumulators/simple_bram_vacc  mask(simple_bram_vacc){vec_len=vector_len, arith_type=Unsigned, n_bits=dout_width, bin_pt=dout_point}
      mask prompts: vec_len "Vector length:"; arith_type "Output type:"; n_bits "Bit width (output):"; bin_pt "Binary point (output):"
      mask help: This simple vector accumulator is based on a shift register. It outputs its previous accumulated vector after a new_acc pulse is received.
      mask init:
      | simple_bram_vacc_init(gcb, ...
      |     'vec_len', vec_len, ...
      |     'arith_type', arith_type, ...
      |     'n_bits', n_bits, ...
      |     'bin_pt', bin_pt);
      mask TypeOptions arith_type: Signed | Unsigned
  valid : Outport
  dout0 : Outport  Port=2
  dout1 : Outport  Port=3
  dout2 : Outport  Port=4
  dout3 : Outport  Port=5
  dout4 : Outport  Port=6
  dout5 : Outport  Port=7
  dout6 : Outport  Port=8
  dout7 : Outport  Port=9
  wires:
  din/1 -> simple_bram_vacc/2(din)
  din3/1 -> simple_bram_vacc3/2(din)
  simple_bram_vacc3/2(valid) -> valid/1
  simple_bram_vacc1/1(dout) -> dout1/1
  simple_bram_vacc2/1(dout) -> dout2/1
  din2/1 -> simple_bram_vacc2/2(din)
  simple_bram_vacc3/1(dout) -> dout3/1
  simple_bram_vacc/1(dout) -> dout0/1
  din1/1 -> simple_bram_vacc1/2(din)
  simple_bram_vacc7/1(dout) -> dout7/1
  simple_bram_vacc6/1(dout) -> dout6/1
  simple_bram_vacc4/1(dout) -> dout4/1
  din7/1 -> simple_bram_vacc7/2(din)
  new_acc/1 -> simple_bram_vacc/1(new_acc), simple_bram_vacc1/1(new_acc), simple_bram_vacc4/1(new_acc), simple_bram_vacc5/1(new_acc), simple_bram_vacc7/1(new_acc), simple_bram_vacc6/1(new_acc), simple_bram_vacc3/1(new_acc), simple_bram_vacc2/1(new_acc)
  din4/1 -> simple_bram_vacc4/2(din)
  din5/1 -> simple_bram_vacc5/2(din)
  simple_bram_vacc5/1(dout) -> dout5/1
  din6/1 -> simple_bram_vacc6/2(din)

== system two_adcs_rfsoc_if_calibrator/calibrator_conv0 ==
  sync_in : Inport
  din0 : Inport  Port=2
  din1 : Inport  Port=3
  din2 : Inport  Port=4
  din3 : Inport  Port=5
  din4 : Inport  Port=6
  din5 : Inport  Port=7
  din6 : Inport  Port=8
  din7 : Inport  Port=9
  Convert{,1..7} (8 alike) : xbsIndex_r4/Convert  gui_display_data_type=Fixed-point  arith_type=Unsigned  n_bits=conv_width  bin_pt=conv_point  float_type=Single  exp_bits=8  fraction_bits=24  quantization=Truncate  overflow=Wrap  en=off  latency=2  pipeline=off  dbl_ovrd=off  block_type=convert
      settings: block_version=11.4
  Shift{1..7,32} (8 alike) : xbsIndex_r4/Shift  shift_dir=Left  shift_bits=shift_val  en=off  latency=0  precision=Full  arith_type="Signed  (2's comp)"  n_bits=16  bin_pt=14  quantization=Truncate  overflow=Wrap  dbl_ovrd=off  block_type=shift
  pipeline : Subsystem  AncestorBlock=casper_library_delays/pipeline  mask(pipeline){csp_latency=2}
      mask prompts: csp_latency "Latency"
      mask help:
      | An explicitly laid-out delay line to avoid mapping to SRL16s
      | for use in pipelining to help achieve timing closure.
      mask init:
      | pipeline_init(gcb,'latency',csp_latency);
      settings: AttributesFormatString=Latency = %<latency>
      mask Display:
      | port_label('input', 1, 'd');
      | port_label('output', 1, 'q');
      | disp(['Z^-', num2str(csp_latency)]);
  sync_out : Outport
  dout0 : Outport  Port=2
  dout1 : Outport  Port=3
  dout2 : Outport  Port=4
  dout3 : Outport  Port=5
  dout4 : Outport  Port=6
  dout5 : Outport  Port=7
  dout6 : Outport  Port=8
  dout7 : Outport  Port=9
  wires:
  din0/1 -> Shift32/1
  Shift32/1 -> Convert/1
  Convert/1 -> dout0/1
  din1/1 -> Shift1/1
  Shift1/1 -> Convert1/1
  Convert1/1 -> dout1/1
  din2/1 -> Shift3/1
  Shift3/1 -> Convert2/1
  Convert2/1 -> dout2/1
  din3/1 -> Shift2/1
  Shift2/1 -> Convert3/1
  Convert3/1 -> dout3/1
  sync_in/1 -> pipeline/1(d)
  pipeline/1(q) -> sync_out/1
  Convert5/1 -> dout5/1
  Shift6/1 -> Convert6/1
  din7/1 -> Shift5/1
  Shift4/1 -> Convert5/1
  Convert6/1 -> dout6/1
  din5/1 -> Shift4/1
  din6/1 -> Shift6/1
  Shift5/1 -> Convert7/1
  Convert7/1 -> dout7/1
  din4/1 -> Shift7/1
  Shift7/1 -> Convert4/1
  Convert4/1 -> dout4/1
